simulink model "simulation_environment_thesis"  (R2024b)
settings: Solver=VariableStepAuto  StartTime=0.0  StopTime=3200  RelTol=1e-3
values from neighbouring files (symbols this model uses):
  A = [1, dt; 0, 1]    [estimate_and_control_fully_actuated_with_current.m]
  A1 = [1, 0, 0, 0, dt, 0 ... (first row only)    [estimate_and_control_fully_actuated_with_current.m]
  alpha = 10    [estimate_and_control_fully_actuated_with_current.m]
  alpha_xy_1 = 1    [estimate_and_control_fully_actuated_with_current.m]
  alpha_z_1 = 5    [estimate_and_control_fully_actuated_with_current.m]
  Az1 = [1, dt ... (first row only)    [estimate_and_control_fully_actuated_with_current.m]
  B = [dt; 0]    [estimate_and_control_fully_actuated_with_current.m]
  B1 = [dt, 0 ... (first row only)    [estimate_and_control_fully_actuated_with_current.m]
  Bz1 = [dt ... (first row only)    [estimate_and_control_fully_actuated_with_current.m]
  C1 = [1, 0, 0, 0, 0, 0 ... (first row only)    [estimate_and_control_fully_actuated_with_current.m]
  circumference_x = min_dist_from_ds*cos(theta)    [estimate_and_control_fully_actuated_with_current.m]
  circumference_y = min_dist_from_ds*sin(theta)    [estimate_and_control_fully_actuated_with_current.m]
  const_current = [-r; -s; 0; 0]    [estimate_and_control_fully_actuated_with_current.m]
  csi_psi_1 = 0.0003    [estimate_and_control_fully_actuated_with_current.m]
  csi_r_1 = 0.0003    [estimate_and_control_fully_actuated_with_current.m]
  csi_vcx_1 = 0.0003    [estimate_and_control_fully_actuated_with_current.m]
  csi_vcy_1 = 0.0003    [estimate_and_control_fully_actuated_with_current.m]
  csi_vcz_1 = 0.005    [estimate_and_control_fully_actuated_with_current.m]
  csi_x_1 = 0.003    [estimate_and_control_fully_actuated_with_current.m]
  csi_y_1 = 0.003    [estimate_and_control_fully_actuated_with_current.m]
  csi_z_1 = 0.005    [estimate_and_control_fully_actuated_with_current.m]
  Cz1 = [1, 0]    [estimate_and_control_fully_actuated_with_current.m]
  d = 1    [estimate_and_control_fully_actuated_with_current.m]
  delta_FOV = pi/7    (= 0.448798950513)    [estimate_and_control_fully_actuated_with_current.m]
  desired_speed = speed_0    [estimate_and_control_fully_actuated_with_current.m]
  dt = 1/Tsim    (= 0.005)    [estimate_and_control_fully_actuated_with_current.m]
  eta1 = deg2rad(0.5)    [estimate_and_control_fully_actuated_with_current.m]
  eta2 = deg2rad(0.5)    [estimate_and_control_fully_actuated_with_current.m]
  eta_psi_1 = 0.003    [estimate_and_control_fully_actuated_with_current.m]
  eta_x_1 = 0.03    [estimate_and_control_fully_actuated_with_current.m]
  eta_y_1 = 0.03    [estimate_and_control_fully_actuated_with_current.m]
  eta_z_1 = 0.005    [estimate_and_control_fully_actuated_with_current.m]
  G = dt*eye(2)    [estimate_and_control_fully_actuated_with_current.m]
  G1 = dt*eye(6)    [estimate_and_control_fully_actuated_with_current.m]
  Gz1 = dt*eye(2)    [estimate_and_control_fully_actuated_with_current.m]
  left_reference = L_x*[cos(NED_psi_ds); sin(NED_psi_ds); 0] + L_y_l*[-sin(NED_psi_ds); cos(NED_psi_ds); 0]    [estimate_and_control_fully_actuated_with_current.m]
  max_dist_from_ship = 60    [estimate_and_control_fully_actuated_with_current.m]
  min_dist_from_ds = 45    [estimate_and_control_fully_actuated_with_current.m]
  mu = -5    [estimate_and_control_fully_actuated_with_current.m]
  NED_psi_ds = -pi/2    (= -1.57079632679)    [estimate_and_control_fully_actuated_with_current.m]
  p_b1 = [-90; 90; 5]    [estimate_and_control_fully_actuated_with_current.m]
  position_t_0 = [0; 0; 0; 0]    [estimate_and_control_fully_actuated_with_current.m]
  psi_P = linspace(0,2*pi,360)'    [estimate_and_control_fully_actuated_with_current.m]
  Q = [csi1_kf^2, 0 ... (first row only)    [estimate_and_control_fully_actuated_with_current.m]
  Q1 = [csi_x_1_kf^2, 0, 0, 0, 0, 0 ... (first row only)    [estimate_and_control_fully_actuated_with_current.m]
  Qz1 = [csi_z_1_kf^2, 0 ... (first row only)    [estimate_and_control_fully_actuated_with_current.m]
  R = [eta1_kf^2, 0 ... (first row only)    [estimate_and_control_fully_actuated_with_current.m]
  R1 = [eta_x_1_kf^2, 0, 0 ... (first row only)    [estimate_and_control_fully_actuated_with_current.m]
  right_reference = L_x*[cos(NED_psi_ds); sin(NED_psi_ds); 0] + L_y_r*[sin(NED_psi_ds); -cos(NED_psi_ds); 0]    [estimate_and_control_fully_actuated_with_current.m]
  Rz1 = eta_z_1_kf^2    (= 1e-06)    [estimate_and_control_fully_actuated_with_current.m]
  speed_0 = [0.1, 0.1, 0, 0]'    [estimate_and_control_fully_actuated_with_current.m]
  tau_heave_sat = 30    [estimate_and_control_fully_actuated_with_current.m]
  tau_surge_sat = 10    [estimate_and_control_fully_actuated_with_current.m]
  tau_sway_sat = 30    [estimate_and_control_fully_actuated_with_current.m]
  tau_yaw_sat = 0.1    [estimate_and_control_fully_actuated_with_current.m]
  var_vm_x = 0.001    [estimate_and_control_fully_actuated_with_current.m]
  var_vm_y = 0.001    [estimate_and_control_fully_actuated_with_current.m]
  var_vm_z = 0.001    [estimate_and_control_fully_actuated_with_current.m]
undefined symbols (not defined in the file or in its folder): A2, alpha2, B2, csi1_2, csi2_2, csi3_2, csi4_2, csi5_2, csi6_2, csi7_2, csi8_2, eta1_2, eta2_2, eta3_2, eta4_2, eta5_2, eta6_2, G2, Q2, R2
libraries: simulink x60, simulink_extras x5
(part 3 of 16)

== system simulation_environment_thesis/toWorkspace blocks 24 ==
  state : Inport
  psi1 : Inport  Port=2
  body_speed_1 : Inport  Port=3
  psi2 : Inport  Port=4
  p1 : Inport  Port=5
  p2 : Inport  Port=6
  speed body2 : Inport  Port=7
  speed body3 : Inport  Port=8
  psi3 : Inport  Port=9
  p3 : Inport  Port=10
  speed_body4 : Inport  Port=11
  psi4 : Inport  Port=12
  p4 : Inport  Port=13
  Demux{,1..2,6} (4 alike) : Demux
  Demux{3..5,7} (4 alike) : Demux  Outputs=3
  Multiport Switch{,1..6} (7 alike) : MultiPortSwitch  Inputs=4  SaturateOnIntegerOverflow=off  InputSameDT=off
  To Workspace : ToWorkspace  VariableName=z  SampleTime=-1
  To Workspace1 : ToWorkspace  VariableName=w  SampleTime=-1
  To Workspace15 : ToWorkspace  VariableName=x  SampleTime=-1
  To Workspace16 : ToWorkspace  VariableName=y  SampleTime=-1
  To Workspace17 : ToWorkspace  VariableName=u  SampleTime=-1
  To Workspace18 : ToWorkspace  VariableName=v  SampleTime=-1
  To Workspace19 : ToWorkspace  VariableName=yaw  SampleTime=-1
  wires:
  Multiport Switch/1 -> To Workspace19/1
  Multiport Switch5/1 -> To Workspace16/1
  Multiport Switch2/1 -> To Workspace18/1
  Multiport Switch1/1 -> To Workspace17/1
  Multiport Switch6/1 -> To Workspace/1
  Multiport Switch3/1 -> To Workspace1/1
  psi1/1 -> Multiport Switch/2
  psi2/1 -> Multiport Switch/3
  psi3/1 -> Multiport Switch/4
  body_speed_1/1 -> Demux/1
  speed body2/1 -> Demux1/1
  speed body3/1 -> Demux2/1
  Demux/1 -> Multiport Switch1/2
  Demux1/1 -> Multiport Switch1/3
  Demux2/1 -> Multiport Switch1/4
  Demux/2 -> Multiport Switch2/2
  Demux1/2 -> Multiport Switch2/3
  Demux2/2 -> Multiport Switch2/4
  Demux/3 -> Multiport Switch3/2
  Demux1/3 -> Multiport Switch3/3
  Demux2/3 -> Multiport Switch3/4
  Demux3/1 -> Multiport Switch4/2
  Demux3/2 -> Multiport Switch5/2
  Demux4/2 -> Multiport Switch5/3
  p3/1 -> Demux5/1
  Demux5/2 -> Multiport Switch5/4
  Demux3/3 -> Multiport Switch6/2
  Demux5/1 -> Multiport Switch4/4
  Multiport Switch4/1 -> To Workspace15/1
  Demux4/3 -> Multiport Switch6/3
  state/1 -> Multiport Switch3/1, Multiport Switch2/1, Multiport Switch1/1, Multiport Switch/1, Multiport Switch6/1, Multiport Switch5/1, Multiport Switch4/1
  p1/1 -> Demux3/1
  p2/1 -> Demux4/1
  Demux4/1 -> Multiport Switch4/3
  Demux5/3 -> Multiport Switch6/4
  speed_body4/1 -> Demux6/1
  psi4/1 -> Multiport Switch/5
  p4/1 -> Demux7/1
  Demux6/1 -> Multiport Switch1/5
  Demux6/2 -> Multiport Switch2/5
  Demux6/3 -> Multiport Switch3/5
  Demux7/1 -> Multiport Switch4/5
  Demux7/2 -> Multiport Switch5/5
  Demux7/3 -> Multiport Switch6/5

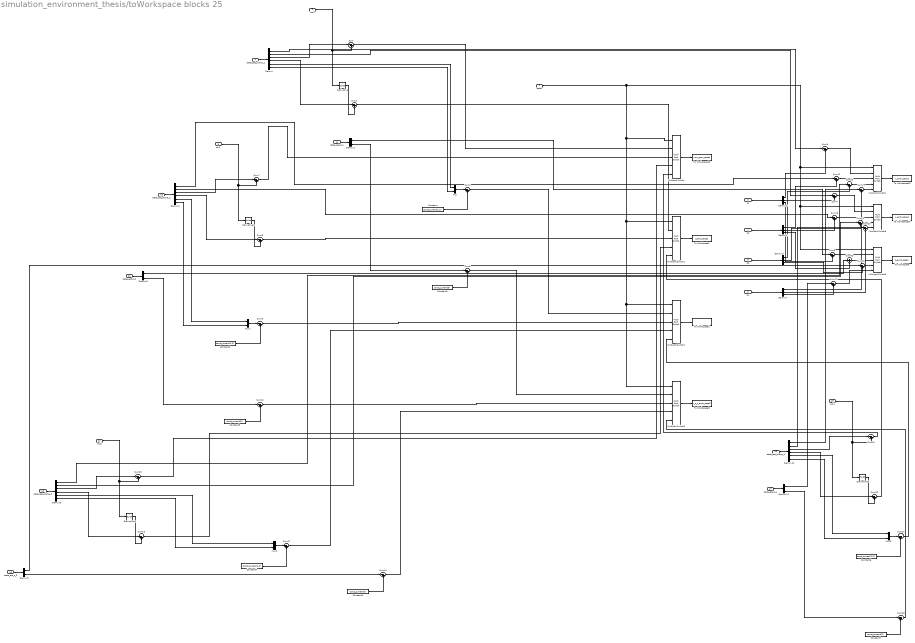
== system simulation_environment_thesis/toWorkspace blocks 25 ==
  state : Inport
  psi1 : Inport  Port=2
  p1 : Inport  Port=3
  p2 : Inport  Port=4
  p3 : Inport  Port=5
  p4 : Inport  Port=6
  state_est_plane_1 : Inport  Port=7
  state_est_z_1 : Inport  Port=8
  psi2 : Inport  Port=9
  state_est_plane_2 : Inport  Port=10
  state_est_z_2 : Inport  Port=11
  psi3 : Inport  Port=12
  state_est_plane_3 : Inport  Port=13
  state_est_z_3 : Inport  Port=14
  psi4 : Inport  Port=15
  state_est_plane_4 : Inport  Port=16
  state_est_z_4 : Inport  Port=17
  Constant{,2,4,6} (4 alike) : Constant  Value=const_current(1:2)
  Constant{1,3,5,7} (4 alike) : Constant  Value=const_current(3)
  Demux{,2,8,10} (4 alike) : Demux  Outputs=6
  Demux{1,6,9,11} (4 alike) : Demux  Outputs=2
  Demux{3..5,7} (4 alike) : Demux  Outputs=3
  Derivative{,1..3} (4 alike) : Derivative
  Multiport Switch{,1..6} (7 alike) : MultiPortSwitch  Inputs=4  SaturateOnIntegerOverflow=off  InputSameDT=off
  Mux{,1..3} (4 alike) : Mux  Inputs=2
  Sum{,1..27} (28 alike) : Sum  Inputs=|-+
  To Workspace : ToWorkspace  VariableName=z_error_case1  SampleTime=-1
  To Workspace1 : ToWorkspace  VariableName=r_error_case1  SampleTime=-1
  To Workspace15 : ToWorkspace  VariableName=x_error_case1  SampleTime=-1
  To Workspace16 : ToWorkspace  VariableName=y_error_case1  SampleTime=-1
  To Workspace19 : ToWorkspace  VariableName=yaw_error_case1  SampleTime=-1
  To Workspace2 : ToWorkspace  VariableName=v_c_x_y_error_case1  SampleTime=-1
  To Workspace3 : ToWorkspace  VariableName=v_c_z_error_case1  SampleTime=-1
  wires:
  Multiport Switch/1 -> To Workspace19/1
  Multiport Switch5/1 -> To Workspace16/1
  Multiport Switch6/1 -> To Workspace/1
  Demux3/1 -> Sum3/2
  p3/1 -> Demux5/1
  Multiport Switch4/1 -> To Workspace15/1
  p1/1 -> Demux3/1
  p2/1 -> Demux4/1
  p4/1 -> Demux7/1
  state_est_plane_1/1 -> Demux/1
  Demux/3 -> Sum/1
  psi1/1 -> Derivative/1, Sum/2
  Sum/1 -> Multiport Switch/2
  Multiport Switch1/1 -> To Workspace1/1
  Derivative/1 -> Sum1/2
  Demux/4 -> Sum1/1
  Sum1/1 -> Multiport Switch1/2
  Sum2/1 -> Multiport Switch2/2
  Multiport Switch2/1 -> To Workspace2/1
  Mux/1 -> Sum2/1
  Demux/5 -> Mux/1
  Demux/6 -> Mux/2
  Constant/1 -> Sum2/2
  Demux/1 -> Sum3/1
  Sum3/1 -> Multiport Switch4/2
  Demux3/2 -> Sum4/2
  Demux/2 -> Sum4/1
  Sum4/1 -> Multiport Switch5/2
  Demux3/3 -> Sum5/2
  state_est_z_1/1 -> Demux1/1
  Demux1/1 -> Sum5/1
  Sum5/1 -> Multiport Switch6/2
  Multiport Switch3/1 -> To Workspace3/1
  Sum6/1 -> Multiport Switch3/2
  state/1 -> Multiport Switch1/1, Multiport Switch3/1, Multiport Switch2/1, Multiport Switch/1, Multiport Switch4/1, Multiport Switch6/1, Multiport Switch5/1
  Constant1/1 -> Sum6/2
  Demux1/2 -> Sum6/1
  Derivative1/1 -> Sum8/2
  Demux6/2 -> Sum10/1
  Demux2/5 -> Mux1/1
  Demux2/4 -> Sum8/1
  Constant3/1 -> Sum10/2
  Demux2/1 -> Sum11/1
  state_est_z_2/1 -> Demux6/1
  Sum7/1 -> Multiport Switch/3
  Demux2/3 -> Sum7/1
  Demux6/1 -> Sum13/1
  Demux2/6 -> Mux1/2
  psi2/1 -> Derivative1/1, Sum7/2
  Constant2/1 -> Sum9/2
  Sum9/1 -> Multiport Switch2/3
  Sum10/1 -> Multiport Switch3/3
  Sum8/1 -> Multiport Switch1/3
  Mux1/1 -> Sum9/1
  state_est_plane_2/1 -> Demux2/1
  Demux2/2 -> Sum12/1
  Sum11/1 -> Multiport Switch4/3
  Demux4/1 -> Sum11/2
  Sum12/1 -> Multiport Switch5/3
  Demux4/2 -> Sum12/2
  Demux4/3 -> Sum13/2
  Sum13/1 -> Multiport Switch6/3
  Constant5/1 -> Sum14/2
  Demux8/4 -> Sum16/1
  Demux9/2 -> Sum14/1
  Demux8/5 -> Mux2/1
  Derivative2/1 -> Sum16/2
  state_est_plane_3/1 -> Demux8/1
  Mux2/1 -> Sum17/1
  Demux8/6 -> Mux2/2
  Constant4/1 -> Sum17/2
  psi3/1 -> Derivative2/1, Sum15/2
  state_est_z_3/1 -> Demux9/1
  Demux8/3 -> Sum15/1
  Demux10/3 -> Sum19/1
  state_est_z_4/1 -> Demux11/1
  psi4/1 -> Derivative3/1, Sum19/2
  Demux10/6 -> Mux3/2
  state_est_plane_4/1 -> Demux10/1
  Constant6/1 -> Sum21/2
  Mux3/1 -> Sum21/1
  Derivative3/1 -> Sum20/2
  Demux10/4 -> Sum20/1
  Demux11/2 -> Sum18/1
  Demux10/5 -> Mux3/1
  Constant7/1 -> Sum18/2
  Sum15/1 -> Multiport Switch/4
  Sum16/1 -> Multiport Switch1/4
  Sum17/1 -> Multiport Switch2/4
  Sum14/1 -> Multiport Switch3/4
  Sum19/1 -> Multiport Switch/5
  Sum20/1 -> Multiport Switch1/5
  Sum21/1 -> Multiport Switch2/5
  Sum18/1 -> Multiport Switch3/5
  Sum22/1 -> Multiport Switch4/4
  Demux5/1 -> Sum22/2
  Demux8/1 -> Sum22/1
  Sum23/1 -> Multiport Switch4/5
  Demux7/1 -> Sum23/2
  Demux10/1 -> Sum23/1
  Sum24/1 -> Multiport Switch5/4
  Demux5/2 -> Sum24/2
  Demux8/2 -> Sum24/1
  Sum25/1 -> Multiport Switch5/5
  Demux7/2 -> Sum25/2
  Demux10/2 -> Sum25/1
  Sum26/1 -> Multiport Switch6/4
  Demux5/3 -> Sum26/2
  Demux9/1 -> Sum26/1
  Sum27/1 -> Multiport Switch6/5
  Demux7/3 -> Sum27/2
  Demux11/1 -> Sum27/1

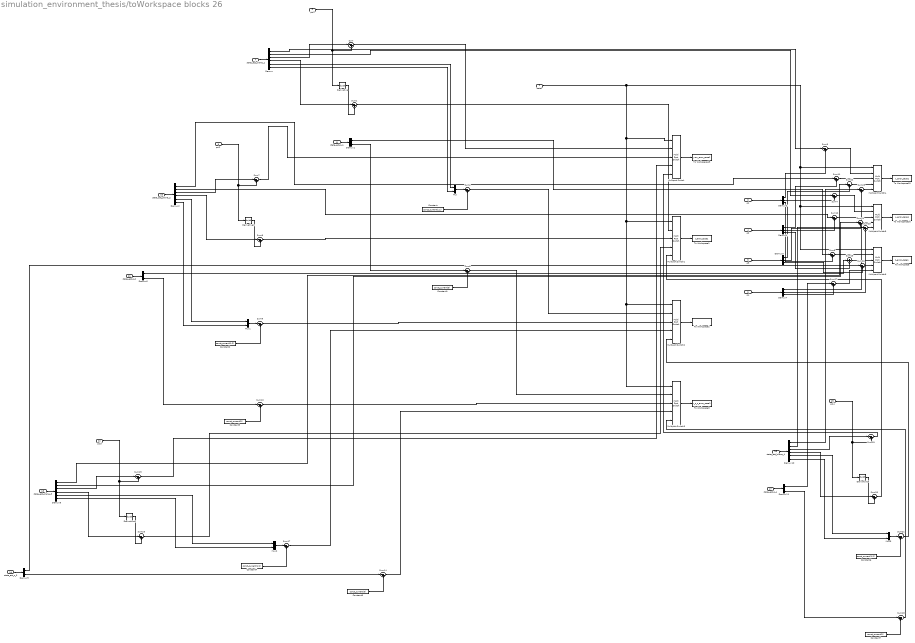
== system simulation_environment_thesis/toWorkspace blocks 26 ==
  state : Inport
  psi1 : Inport  Port=2
  p1 : Inport  Port=3
  p2 : Inport  Port=4
  p3 : Inport  Port=5
  p4 : Inport  Port=6
  state_est_plane_1 : Inport  Port=7
  state_est_z_1 : Inport  Port=8
  psi2 : Inport  Port=9
  state_est_plane_2 : Inport  Port=10
  state_est_z_2 : Inport  Port=11
  psi3 : Inport  Port=12
  state_est_plane_3 : Inport  Port=13
  state_est_z_3 : Inport  Port=14
  psi4 : Inport  Port=15
  state_est_plane_4 : Inport  Port=16
  state_est_z_4 : Inport  Port=17
  Constant{,2,4,6} (4 alike) : Constant  Value=const_current(1:2)
  Constant{1,3,5,7} (4 alike) : Constant  Value=const_current(3)
  Demux{,2,8,10} (4 alike) : Demux  Outputs=6
  Demux{1,6,9,11} (4 alike) : Demux  Outputs=2
  Demux{3..5,7} (4 alike) : Demux  Outputs=3
  Derivative{,1..3} (4 alike) : Derivative
  Multiport Switch{,1..6} (7 alike) : MultiPortSwitch  Inputs=4  SaturateOnIntegerOverflow=off  InputSameDT=off
  Mux{,1..3} (4 alike) : Mux  Inputs=2
  Sum{,1..27} (28 alike) : Sum  Inputs=|-+
  To Workspace : ToWorkspace  VariableName=z_error_case1  SampleTime=-1
  To Workspace1 : ToWorkspace  VariableName=r_error_case1  SampleTime=-1
  To Workspace15 : ToWorkspace  VariableName=x_error_case1  SampleTime=-1
  To Workspace16 : ToWorkspace  VariableName=y_error_case1  SampleTime=-1
  To Workspace19 : ToWorkspace  VariableName=yaw_error_case1  SampleTime=-1
  To Workspace2 : ToWorkspace  VariableName=v_c_x_y_error_case1  SampleTime=-1
  To Workspace3 : ToWorkspace  VariableName=v_c_z_error_case1  SampleTime=-1
  wires:
  Multiport Switch/1 -> To Workspace19/1
  Multiport Switch5/1 -> To Workspace16/1
  Multiport Switch6/1 -> To Workspace/1
  Demux3/1 -> Sum3/2
  p3/1 -> Demux5/1
  Multiport Switch4/1 -> To Workspace15/1
  p1/1 -> Demux3/1
  p2/1 -> Demux4/1
  p4/1 -> Demux7/1
  state_est_plane_1/1 -> Demux/1
  Demux/3 -> Sum/1
  psi1/1 -> Derivative/1, Sum/2
  Sum/1 -> Multiport Switch/2
  Multiport Switch1/1 -> To Workspace1/1
  Derivative/1 -> Sum1/2
  Demux/4 -> Sum1/1
  Sum1/1 -> Multiport Switch1/2
  Sum2/1 -> Multiport Switch2/2
  Multiport Switch2/1 -> To Workspace2/1
  Mux/1 -> Sum2/1
  Demux/5 -> Mux/1
  Demux/6 -> Mux/2
  Constant/1 -> Sum2/2
  Demux/1 -> Sum3/1
  Sum3/1 -> Multiport Switch4/2
  Demux3/2 -> Sum4/2
  Demux/2 -> Sum4/1
  Sum4/1 -> Multiport Switch5/2
  Demux3/3 -> Sum5/2
  state_est_z_1/1 -> Demux1/1
  Demux1/1 -> Sum5/1
  Sum5/1 -> Multiport Switch6/2
  Multiport Switch3/1 -> To Workspace3/1
  Sum6/1 -> Multiport Switch3/2
  state/1 -> Multiport Switch1/1, Multiport Switch3/1, Multiport Switch2/1, Multiport Switch/1, Multiport Switch4/1, Multiport Switch6/1, Multiport Switch5/1
  Constant1/1 -> Sum6/2
  Demux1/2 -> Sum6/1
  Derivative1/1 -> Sum8/2
  Demux6/2 -> Sum10/1
  Demux2/5 -> Mux1/1
  Demux2/4 -> Sum8/1
  Constant3/1 -> Sum10/2
  Demux2/1 -> Sum11/1
  state_est_z_2/1 -> Demux6/1
  Sum7/1 -> Multiport Switch/3
  Demux2/3 -> Sum7/1
  Demux6/1 -> Sum13/1
  Demux2/6 -> Mux1/2
  psi2/1 -> Derivative1/1, Sum7/2
  Constant2/1 -> Sum9/2
  Sum9/1 -> Multiport Switch2/3
  Sum10/1 -> Multiport Switch3/3
  Sum8/1 -> Multiport Switch1/3
  Mux1/1 -> Sum9/1
  state_est_plane_2/1 -> Demux2/1
  Demux2/2 -> Sum12/1
  Sum11/1 -> Multiport Switch4/3
  Demux4/1 -> Sum11/2
  Sum12/1 -> Multiport Switch5/3
  Demux4/2 -> Sum12/2
  Demux4/3 -> Sum13/2
  Sum13/1 -> Multiport Switch6/3
  Constant5/1 -> Sum14/2
  Demux8/4 -> Sum16/1
  Demux9/2 -> Sum14/1
  Demux8/5 -> Mux2/1
  Derivative2/1 -> Sum16/2
  state_est_plane_3/1 -> Demux8/1
  Mux2/1 -> Sum17/1
  Demux8/6 -> Mux2/2
  Constant4/1 -> Sum17/2
  psi3/1 -> Derivative2/1, Sum15/2
  state_est_z_3/1 -> Demux9/1
  Demux8/3 -> Sum15/1
  Demux10/3 -> Sum19/1
  state_est_z_4/1 -> Demux11/1
  psi4/1 -> Derivative3/1, Sum19/2
  Demux10/6 -> Mux3/2
  state_est_plane_4/1 -> Demux10/1
  Constant6/1 -> Sum21/2
  Mux3/1 -> Sum21/1
  Derivative3/1 -> Sum20/2
  Demux10/4 -> Sum20/1
  Demux11/2 -> Sum18/1
  Demux10/5 -> Mux3/1
  Constant7/1 -> Sum18/2
  Sum15/1 -> Multiport Switch/4
  Sum16/1 -> Multiport Switch1/4
  Sum17/1 -> Multiport Switch2/4
  Sum14/1 -> Multiport Switch3/4
  Sum19/1 -> Multiport Switch/5
  Sum20/1 -> Multiport Switch1/5
  Sum21/1 -> Multiport Switch2/5
  Sum18/1 -> Multiport Switch3/5
  Sum22/1 -> Multiport Switch4/4
  Demux5/1 -> Sum22/2
  Demux8/1 -> Sum22/1
  Sum23/1 -> Multiport Switch4/5
  Demux7/1 -> Sum23/2
  Demux10/1 -> Sum23/1
  Sum24/1 -> Multiport Switch5/4
  Demux5/2 -> Sum24/2
  Demux8/2 -> Sum24/1
  Sum25/1 -> Multiport Switch5/5
  Demux7/2 -> Sum25/2
  Demux10/2 -> Sum25/1
  Sum26/1 -> Multiport Switch6/4
  Demux5/3 -> Sum26/2
  Demux9/1 -> Sum26/1
  Sum27/1 -> Multiport Switch6/5
  Demux7/3 -> Sum27/2
  Demux11/1 -> Sum27/1

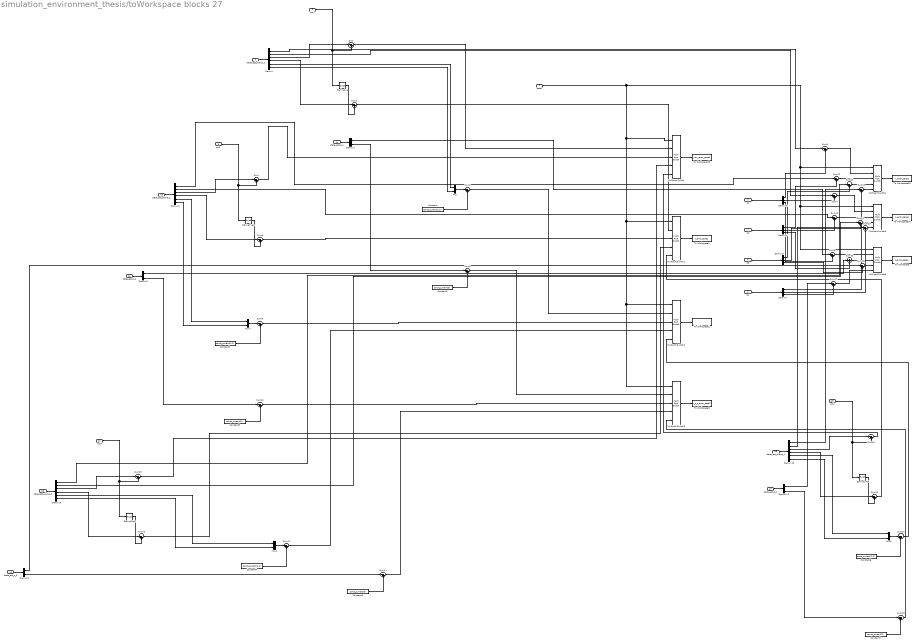
== system simulation_environment_thesis/toWorkspace blocks 27 ==
  state : Inport
  psi1 : Inport  Port=2
  p1 : Inport  Port=3
  p2 : Inport  Port=4
  p3 : Inport  Port=5
  p4 : Inport  Port=6
  state_est_plane_1 : Inport  Port=7
  state_est_z_1 : Inport  Port=8
  psi2 : Inport  Port=9
  state_est_plane_2 : Inport  Port=10
  state_est_z_2 : Inport  Port=11
  psi3 : Inport  Port=12
  state_est_plane_3 : Inport  Port=13
  state_est_z_3 : Inport  Port=14
  psi4 : Inport  Port=15
  state_est_plane_4 : Inport  Port=16
  state_est_z_4 : Inport  Port=17
  Constant{,2,4,6} (4 alike) : Constant  Value=const_current(1:2)
  Constant{1,3,5,7} (4 alike) : Constant  Value=const_current(3)
  Demux{,2,8,10} (4 alike) : Demux  Outputs=6
  Demux{1,6,9,11} (4 alike) : Demux  Outputs=2
  Demux{3..5,7} (4 alike) : Demux  Outputs=3
  Derivative{,1..3} (4 alike) : Derivative
  Multiport Switch{,1..6} (7 alike) : MultiPortSwitch  Inputs=4  SaturateOnIntegerOverflow=off  InputSameDT=off
  Mux{,1..3} (4 alike) : Mux  Inputs=2
  Sum{,1..27} (28 alike) : Sum  Inputs=|-+
  To Workspace : ToWorkspace  VariableName=z_error_case1  SampleTime=-1
  To Workspace1 : ToWorkspace  VariableName=r_error_case1  SampleTime=-1
  To Workspace15 : ToWorkspace  VariableName=x_error_case1  SampleTime=-1
  To Workspace16 : ToWorkspace  VariableName=y_error_case1  SampleTime=-1
  To Workspace19 : ToWorkspace  VariableName=yaw_error_case1  SampleTime=-1
  To Workspace2 : ToWorkspace  VariableName=v_c_x_y_error_case1  SampleTime=-1
  To Workspace3 : ToWorkspace  VariableName=v_c_z_error_case1  SampleTime=-1
  wires:
  Multiport Switch/1 -> To Workspace19/1
  Multiport Switch5/1 -> To Workspace16/1
  Multiport Switch6/1 -> To Workspace/1
  Demux3/1 -> Sum3/2
  p3/1 -> Demux5/1
  Multiport Switch4/1 -> To Workspace15/1
  p1/1 -> Demux3/1
  p2/1 -> Demux4/1
  p4/1 -> Demux7/1
  state_est_plane_1/1 -> Demux/1
  Demux/3 -> Sum/1
  psi1/1 -> Derivative/1, Sum/2
  Sum/1 -> Multiport Switch/2
  Multiport Switch1/1 -> To Workspace1/1
  Derivative/1 -> Sum1/2
  Demux/4 -> Sum1/1
  Sum1/1 -> Multiport Switch1/2
  Sum2/1 -> Multiport Switch2/2
  Multiport Switch2/1 -> To Workspace2/1
  Mux/1 -> Sum2/1
  Demux/5 -> Mux/1
  Demux/6 -> Mux/2
  Constant/1 -> Sum2/2
  Demux/1 -> Sum3/1
  Sum3/1 -> Multiport Switch4/2
  Demux3/2 -> Sum4/2
  Demux/2 -> Sum4/1
  Sum4/1 -> Multiport Switch5/2
  Demux3/3 -> Sum5/2
  state_est_z_1/1 -> Demux1/1
  Demux1/1 -> Sum5/1
  Sum5/1 -> Multiport Switch6/2
  Multiport Switch3/1 -> To Workspace3/1
  Sum6/1 -> Multiport Switch3/2
  state/1 -> Multiport Switch1/1, Multiport Switch3/1, Multiport Switch2/1, Multiport Switch/1, Multiport Switch4/1, Multiport Switch6/1, Multiport Switch5/1
  Constant1/1 -> Sum6/2
  Demux1/2 -> Sum6/1
  Derivative1/1 -> Sum8/2
  Demux6/2 -> Sum10/1
  Demux2/5 -> Mux1/1
  Demux2/4 -> Sum8/1
  Constant3/1 -> Sum10/2
  Demux2/1 -> Sum11/1
  state_est_z_2/1 -> Demux6/1
  Sum7/1 -> Multiport Switch/3
  Demux2/3 -> Sum7/1
  Demux6/1 -> Sum13/1
  Demux2/6 -> Mux1/2
  psi2/1 -> Derivative1/1, Sum7/2
  Constant2/1 -> Sum9/2
  Sum9/1 -> Multiport Switch2/3
  Sum10/1 -> Multiport Switch3/3
  Sum8/1 -> Multiport Switch1/3
  Mux1/1 -> Sum9/1
  state_est_plane_2/1 -> Demux2/1
  Demux2/2 -> Sum12/1
  Sum11/1 -> Multiport Switch4/3
  Demux4/1 -> Sum11/2
  Sum12/1 -> Multiport Switch5/3
  Demux4/2 -> Sum12/2
  Demux4/3 -> Sum13/2
  Sum13/1 -> Multiport Switch6/3
  Constant5/1 -> Sum14/2
  Demux8/4 -> Sum16/1
  Demux9/2 -> Sum14/1
  Demux8/5 -> Mux2/1
  Derivative2/1 -> Sum16/2
  state_est_plane_3/1 -> Demux8/1
  Mux2/1 -> Sum17/1
  Demux8/6 -> Mux2/2
  Constant4/1 -> Sum17/2
  psi3/1 -> Derivative2/1, Sum15/2
  state_est_z_3/1 -> Demux9/1
  Demux8/3 -> Sum15/1
  Demux10/3 -> Sum19/1
  state_est_z_4/1 -> Demux11/1
  psi4/1 -> Derivative3/1, Sum19/2
  Demux10/6 -> Mux3/2
  state_est_plane_4/1 -> Demux10/1
  Constant6/1 -> Sum21/2
  Mux3/1 -> Sum21/1
  Derivative3/1 -> Sum20/2
  Demux10/4 -> Sum20/1
  Demux11/2 -> Sum18/1
  Demux10/5 -> Mux3/1
  Constant7/1 -> Sum18/2
  Sum15/1 -> Multiport Switch/4
  Sum16/1 -> Multiport Switch1/4
  Sum17/1 -> Multiport Switch2/4
  Sum14/1 -> Multiport Switch3/4
  Sum19/1 -> Multiport Switch/5
  Sum20/1 -> Multiport Switch1/5
  Sum21/1 -> Multiport Switch2/5
  Sum18/1 -> Multiport Switch3/5
  Sum22/1 -> Multiport Switch4/4
  Demux5/1 -> Sum22/2
  Demux8/1 -> Sum22/1
  Sum23/1 -> Multiport Switch4/5
  Demux7/1 -> Sum23/2
  Demux10/1 -> Sum23/1
  Sum24/1 -> Multiport Switch5/4
  Demux5/2 -> Sum24/2
  Demux8/2 -> Sum24/1
  Sum25/1 -> Multiport Switch5/5
  Demux7/2 -> Sum25/2
  Demux10/2 -> Sum25/1
  Sum26/1 -> Multiport Switch6/4
  Demux5/3 -> Sum26/2
  Demux9/1 -> Sum26/1
  Sum27/1 -> Multiport Switch6/5
  Demux7/3 -> Sum27/2
  Demux11/1 -> Sum27/1

== system simulation_environment_thesis/toWorkspace blocks 29 ==
  state : Inport
  psi1 : Inport  Port=2
  body_speed_1 : Inport  Port=3
  psi2 : Inport  Port=4
  p1 : Inport  Port=5
  p2 : Inport  Port=6
  speed body2 : Inport  Port=7
  speed body3 : Inport  Port=8
  psi3 : Inport  Port=9
  p3 : Inport  Port=10
  speed_body4 : Inport  Port=11
  psi4 : Inport  Port=12
  p4 : Inport  Port=13
  Demux{,1..2,6} (4 alike) : Demux
  Multiport Switch{,1..4,6} (6 alike) : MultiPortSwitch  Inputs=4  SaturateOnIntegerOverflow=off  InputSameDT=off
  To Workspace : ToWorkspace  VariableName=z_error  SampleTime=-1
  To Workspace1 : ToWorkspace  VariableName=w_error  SampleTime=-1
  To Workspace17 : ToWorkspace  VariableName=u_error  SampleTime=-1
  To Workspace18 : ToWorkspace  VariableName=v_error  SampleTime=-1
  To Workspace19 : ToWorkspace  VariableName=yaw_error  SampleTime=-1
  To Workspace2 : ToWorkspace  VariableName=r_error  SampleTime=-1
  wires:
  Multiport Switch/1 -> To Workspace19/1
  Multiport Switch2/1 -> To Workspace18/1
  Multiport Switch1/1 -> To Workspace17/1
  Multiport Switch6/1 -> To Workspace/1
  Multiport Switch3/1 -> To Workspace1/1
  psi1/1 -> Multiport Switch/2
  psi2/1 -> Multiport Switch/3
  psi3/1 -> Multiport Switch/4
  body_speed_1/1 -> Demux/1
  speed body2/1 -> Demux1/1
  speed body3/1 -> Demux2/1
  Demux/1 -> Multiport Switch1/2
  Demux1/1 -> Multiport Switch1/3
  Demux2/1 -> Multiport Switch1/4
  Demux/2 -> Multiport Switch2/2
  Demux1/2 -> Multiport Switch2/3
  Demux2/2 -> Multiport Switch2/4
  Demux/3 -> Multiport Switch3/2
  Demux1/3 -> Multiport Switch3/3
  Demux2/3 -> Multiport Switch3/4
  p1/1 -> Multiport Switch6/2
  p2/1 -> Multiport Switch6/3
  state/1 -> Multiport Switch4/1, Multiport Switch3/1, Multiport Switch2/1, Multiport Switch1/1, Multiport Switch/1, Multiport Switch6/1
  p3/1 -> Multiport Switch6/4
  speed_body4/1 -> Demux6/1
  psi4/1 -> Multiport Switch/5
  Demux6/1 -> Multiport Switch1/5
  Demux6/2 -> Multiport Switch2/5
  Demux6/3 -> Multiport Switch3/5
  p4/1 -> Multiport Switch6/5
  Multiport Switch4/1 -> To Workspace2/1
  Demux/4 -> Multiport Switch4/2
  Demux1/4 -> Multiport Switch4/3
  Demux2/4 -> Multiport Switch4/4
  Demux6/4 -> Multiport Switch4/5

== system simulation_environment_thesis/toWorkspace blocks 30 ==
  state : Inport
  psi1 : Inport  Port=2
  body_speed_1 : Inport  Port=3
  psi2 : Inport  Port=4
  p1 : Inport  Port=5
  p2 : Inport  Port=6
  speed body2 : Inport  Port=7
  speed body3 : Inport  Port=8
  psi3 : Inport  Port=9
  p3 : Inport  Port=10
  speed_body4 : Inport  Port=11
  psi4 : Inport  Port=12
  p4 : Inport  Port=13
  Demux{,1..2,6} (4 alike) : Demux
  Multiport Switch{,1..4,6} (6 alike) : MultiPortSwitch  Inputs=4  SaturateOnIntegerOverflow=off  InputSameDT=off
  To Workspace : ToWorkspace  VariableName=z_error  SampleTime=-1
  To Workspace1 : ToWorkspace  VariableName=w_error  SampleTime=-1
  To Workspace17 : ToWorkspace  VariableName=u_error  SampleTime=-1
  To Workspace18 : ToWorkspace  VariableName=v_error  SampleTime=-1
  To Workspace19 : ToWorkspace  VariableName=yaw_error  SampleTime=-1
  To Workspace2 : ToWorkspace  VariableName=r_error  SampleTime=-1
  wires:
  Multiport Switch/1 -> To Workspace19/1
  Multiport Switch2/1 -> To Workspace18/1
  Multiport Switch1/1 -> To Workspace17/1
  Multiport Switch6/1 -> To Workspace/1
  Multiport Switch3/1 -> To Workspace1/1
  psi1/1 -> Multiport Switch/2
  psi2/1 -> Multiport Switch/3
  psi3/1 -> Multiport Switch/4
  body_speed_1/1 -> Demux/1
  speed body2/1 -> Demux1/1
  speed body3/1 -> Demux2/1
  Demux/1 -> Multiport Switch1/2
  Demux1/1 -> Multiport Switch1/3
  Demux2/1 -> Multiport Switch1/4
  Demux/2 -> Multiport Switch2/2
  Demux1/2 -> Multiport Switch2/3
  Demux2/2 -> Multiport Switch2/4
  Demux/3 -> Multiport Switch3/2
  Demux1/3 -> Multiport Switch3/3
  Demux2/3 -> Multiport Switch3/4
  p1/1 -> Multiport Switch6/2
  p2/1 -> Multiport Switch6/3
  state/1 -> Multiport Switch4/1, Multiport Switch3/1, Multiport Switch2/1, Multiport Switch1/1, Multiport Switch/1, Multiport Switch6/1
  p3/1 -> Multiport Switch6/4
  speed_body4/1 -> Demux6/1
  psi4/1 -> Multiport Switch/5
  Demux6/1 -> Multiport Switch1/5
  Demux6/2 -> Multiport Switch2/5
  Demux6/3 -> Multiport Switch3/5
  p4/1 -> Multiport Switch6/5
  Multiport Switch4/1 -> To Workspace2/1
  Demux/4 -> Multiport Switch4/2
  Demux1/4 -> Multiport Switch4/3
  Demux2/4 -> Multiport Switch4/4
  Demux6/4 -> Multiport Switch4/5

== system simulation_environment_thesis/toWorkspace blocks 31 ==
  state : Inport
  psi1 : Inport  Port=2
  body_speed_1 : Inport  Port=3
  psi2 : Inport  Port=4
  p1 : Inport  Port=5
  p2 : Inport  Port=6
  speed body2 : Inport  Port=7
  speed body3 : Inport  Port=8
  psi3 : Inport  Port=9
  p3 : Inport  Port=10
  speed_body4 : Inport  Port=11
  psi4 : Inport  Port=12
  p4 : Inport  Port=13
  Demux{,1..2,6} (4 alike) : Demux
  Multiport Switch{,1..4,6} (6 alike) : MultiPortSwitch  Inputs=4  SaturateOnIntegerOverflow=off  InputSameDT=off
  To Workspace : ToWorkspace  VariableName=z_error  SampleTime=-1
  To Workspace1 : ToWorkspace  VariableName=w_error  SampleTime=-1
  To Workspace17 : ToWorkspace  VariableName=u_error  SampleTime=-1
  To Workspace18 : ToWorkspace  VariableName=v_error  SampleTime=-1
  To Workspace19 : ToWorkspace  VariableName=yaw_error  SampleTime=-1
  To Workspace2 : ToWorkspace  VariableName=r_error  SampleTime=-1
  wires:
  Multiport Switch/1 -> To Workspace19/1
  Multiport Switch2/1 -> To Workspace18/1
  Multiport Switch1/1 -> To Workspace17/1
  Multiport Switch6/1 -> To Workspace/1
  Multiport Switch3/1 -> To Workspace1/1
  psi1/1 -> Multiport Switch/2
  psi2/1 -> Multiport Switch/3
  psi3/1 -> Multiport Switch/4
  body_speed_1/1 -> Demux/1
  speed body2/1 -> Demux1/1
  speed body3/1 -> Demux2/1
  Demux/1 -> Multiport Switch1/2
  Demux1/1 -> Multiport Switch1/3
  Demux2/1 -> Multiport Switch1/4
  Demux/2 -> Multiport Switch2/2
  Demux1/2 -> Multiport Switch2/3
  Demux2/2 -> Multiport Switch2/4
  Demux/3 -> Multiport Switch3/2
  Demux1/3 -> Multiport Switch3/3
  Demux2/3 -> Multiport Switch3/4
  p1/1 -> Multiport Switch6/2
  p2/1 -> Multiport Switch6/3
  state/1 -> Multiport Switch4/1, Multiport Switch3/1, Multiport Switch2/1, Multiport Switch1/1, Multiport Switch/1, Multiport Switch6/1
  p3/1 -> Multiport Switch6/4
  speed_body4/1 -> Demux6/1
  psi4/1 -> Multiport Switch/5
  Demux6/1 -> Multiport Switch1/5
  Demux6/2 -> Multiport Switch2/5
  Demux6/3 -> Multiport Switch3/5
  p4/1 -> Multiport Switch6/5
  Multiport Switch4/1 -> To Workspace2/1
  Demux/4 -> Multiport Switch4/2
  Demux1/4 -> Multiport Switch4/3
  Demux2/4 -> Multiport Switch4/4
  Demux6/4 -> Multiport Switch4/5

== system simulation_environment_thesis/PHASE 1  /CONTROLLER1 ==
  v_err : Inport
  speed_0 : Inport  Port=2  PortDimensions=[4,1]
  yaw_err : Inport  Port=3
  z_err : Inport  Port=4
  ANTIWINDUP SURGE : Subsystem
  ANTIWINDUP SWAY : Subsystem
  ANTIWINDUP_HEAVE : Subsystem
  ANTIWINDUP_YAW : Subsystem
  Demux : Demux
  MATLAB Function3 : MATLAB Function  TreatAsAtomicUnit=on
  Mux : Mux
  tau : Outport
  wires:
  ANTIWINDUP SURGE/1(tau_u) -> Mux/1
  ANTIWINDUP_HEAVE/1(tau_w) -> Mux/3
  MATLAB Function3/1(K_i_u) -> ANTIWINDUP SURGE/2(K_int)
  MATLAB Function3/3(K_t_u) -> ANTIWINDUP SURGE/4(K_t_u)
  MATLAB Function3/2(K_p_u) -> ANTIWINDUP SURGE/3(K_prop)
  speed_0/1 -> MATLAB Function3/1(speed_0)
  Demux/1 -> ANTIWINDUP SURGE/1(in)
  ANTIWINDUP SWAY/1(tau_v) -> Mux/2
  v_err/1 -> Demux/1
  Mux/1 -> tau/1
  Demux/2 -> ANTIWINDUP SWAY/1(in)
  MATLAB Function3/4(K_i_v) -> ANTIWINDUP SWAY/2(K_int)
  MATLAB Function3/5(K_p_v) -> ANTIWINDUP SWAY/3(K_prop)
  MATLAB Function3/6(K_t_v) -> ANTIWINDUP SWAY/4(K_t_v)
  ANTIWINDUP_YAW/1(tau_psi) -> Mux/4
  MATLAB Function3/14(K_t_psi) -> ANTIWINDUP_YAW/3(K_t_psi)
  MATLAB Function3/13(K_d_psi) -> ANTIWINDUP_YAW/6(K_der)
  Demux/4 -> ANTIWINDUP_YAW/5(in)
  MATLAB Function3/12(K_p_psi) -> ANTIWINDUP_YAW/2(K_prop)
  MATLAB Function3/11(K_i_psi) -> ANTIWINDUP_YAW/1(K_int)
  yaw_err/1 -> ANTIWINDUP_YAW/4(in1)
  MATLAB Function3/7(K_i_w) -> ANTIWINDUP_HEAVE/1(K_int)
  MATLAB Function3/8(K_p_w) -> ANTIWINDUP_HEAVE/2(K_prop)
  MATLAB Function3/10(K_t_w) -> ANTIWINDUP_HEAVE/3(K_t_w)
  MATLAB Function3/9(K_d_w) -> ANTIWINDUP_HEAVE/6(K_der)
  z_err/1 -> ANTIWINDUP_HEAVE/4(z_err)
  Demux/3 -> ANTIWINDUP_HEAVE/5(w_err)

== system simulation_environment_thesis/PHASE 1  /REFERENCE GENERATOR ==
  p : Inport
  v_c : Inport  Port=2
  Constant : Constant  Value=p_b1
  Constant1 : Constant  Value=max_dist_from_ship
  Constant3 : Constant  Value=NED_psi_ds + pi
  Constant4 : Constant  Value=speed_0
  MATLAB Function1 : MATLAB Function  TreatAsAtomicUnit=on
  MATLAB Function7 : MATLAB Function  TreatAsAtomicUnit=on
  Memory : Memory
  speed : Outport
  yaw_dock : Outport  Port=2
  stop_flag : Outport  Port=3
  wires:
  MATLAB Function7/2(stop_flag) -> stop_flag/1, Memory/1
  Constant/1 -> MATLAB Function7/4(beacon)
  Memory/1 -> MATLAB Function7/1("stop_flag ")
  Constant3/1 -> MATLAB Function1/1("angle ")
  Constant1/1 -> MATLAB Function7/7(max_distance_from_ship)
  MATLAB Function1/1(angle) -> yaw_dock/1, MATLAB Function7/5(yaw_dock)
  Constant4/1 -> MATLAB Function7/6(speed_0)
  v_c/1 -> MATLAB Function7/2(v_c)
  p/1 -> MATLAB Function7/3(p)
  MATLAB Function7/1(speed) -> speed/1

== system simulation_environment_thesis/PHASE 1  /dynamic + kinematic models1 ==
  note: body frame
  note: ship frame
  note: ship frame
  tau : Inport
  ref speed : Inport  Port=2
  yaw prev : Inport  Port=3
  Constant : Constant  Value=0
  Demux3 : Demux
  Integrator : Integrator
  Integrator1 : Integrator  InitialCondition=const_current
  Integrator12 : Integrator  InitialConditionSource=external
  Integrator7 : Integrator  InitialConditionSource=external  InitialCondition=speed_0  UpperSaturationLimit=10^3  LowerSaturationLimit=-10^3
  MATLAB Function : MATLAB Function  TreatAsAtomicUnit=on
  MATLAB Function7 : MATLAB Function  TreatAsAtomicUnit=on
  MATLAB Function9 : MATLAB Function  TreatAsAtomicUnit=on
  Mux : Mux  Inputs=3
  Mux1 : Mux  Inputs=2
  Mux2 : Mux
  Random Number : RandomNumber  Variance=csi_x_1^2  Seed=75580913  SampleTime=dt
  Random Number1 : RandomNumber  Variance=csi_z_1^2  Seed=2046867146  SampleTime=dt
  Random Number10 : RandomNumber  Variance=csi_r_1^2  Seed=465862562  SampleTime=dt
  Random Number2 : RandomNumber  Variance=csi_vcz_1^2  Seed=1944131077  SampleTime=dt
  Random Number5 : RandomNumber  Variance=csi_y_1^2  Seed=116106138  SampleTime=dt
  Random Number6 : RandomNumber  Variance=csi_vcx_1^2  Seed=2146375792  SampleTime=dt
  Random Number7 : RandomNumber  Variance=csi_vcy_1^2  Seed=868631178  SampleTime=dt
  Random Number9 : RandomNumber  Variance=csi_psi_1^2  Seed=739183364  SampleTime=dt
  Selector : Selector  InputPortWidth=4  IndexOptions=Index vector (dialog)  Indices=[1 2 3]  OutputSizes=1
  Selector1 : Selector  InputPortWidth=4  IndexOptions=Index vector (dialog)  Indices=[4]  OutputSizes=1
  Selector2 : Selector  InputPortWidth=4  IndexOptions=Index vector (dialog)  Indices=[1 2 3]  OutputSizes=1
  Sum{,1..2,5} (4 alike) : Sum  Inputs=|++
  Sum4 : Sum  Inputs=|+-
  Unit Delay2 : UnitDelay  InitialCondition=position_t_0(4)  SampleTime=-1  HasFrameUpgradeWarning=on
  error speed : Outport
  v_tot inert : Outport  Port=2
  psi : Outport  Port=3
  p : Outport  Port=4
  body speed : Outport  Port=5
  wires:
  Integrator1/1 -> Sum5/2
  MATLAB Function7/1(speed_dot) -> Selector2/1, Selector1/1
  ref speed/1 -> Integrator7/2, Sum4/1
  Unit Delay2/1 -> Sum1/1, MATLAB Function9/1(yaw)
  Integrator12/1 -> Demux3/1
  MATLAB Function9/1(eta_dot) -> Integrator12/1, Sum5/1
  Sum4/1 -> error speed/1
  Sum5/1 -> Selector/1, v_tot inert/1
  yaw prev/1 -> Unit Delay2/1
  Integrator7/1 -> MATLAB Function7/1(speed), MATLAB Function9/2(speed), Sum4/2, body speed/1
  Demux3/4 -> MATLAB Function/1("angle ")
  tau/1 -> MATLAB Function7/2(tau)
  Integrator/1 -> p/1
  MATLAB Function/1(angle) -> psi/1
  Random Number10/1 -> Sum2/2
  Random Number7/1 -> Mux2/2
  Random Number6/1 -> Mux2/1
  Random Number9/1 -> Sum1/2
  Random Number/1 -> Mux/1
  Random Number5/1 -> Mux/2
  Sum/1 -> Integrator/1
  Mux/1 -> Sum/2
  Sum1/1 -> Integrator12/2
  Random Number1/1 -> Mux/3
  Selector1/1 -> Sum2/1
  Sum2/1 -> Mux1/2
  Selector2/1 -> Mux1/1
  Mux1/1 -> Integrator7/1
  Random Number2/1 -> Mux2/3
  Mux2/1 -> Integrator1/1
  Constant/1 -> Mux2/4
  Selector/1 -> Sum/1

== system simulation_environment_thesis/PHASE 1  /x,y,psi,r,vc estimate, XY PLANE from x,y,psi ==
  note: x_k_kprev
  note: x_knext_k
  note: ship frame
  note: [x
      | y]
  note: v
  note: doppler measurement noise
  note: u_k = v_m_k
  p : Inport
  inert_vel : Inport  Port=2
  psi : Inport  Port=3
  Constant : Constant  Value=C1
  Constant1 : Constant  Value=p_b1(1:2)
  Constant10 : Constant  Value=B1
  Constant12 : Constant  Value=const_current(1:2,1)  VectorParams1D=off
  Constant13 : Constant  Value=R1
  Constant14 : Constant  Value=A1
  Constant15 : Constant  Value=Q1
  Constant16 : Constant  Value=G1
  Constant9 : Constant  Value=A1  VectorParams1D=off
  Derivative : Derivative
  MATLAB Function : MATLAB Function  TreatAsAtomicUnit=on
  MATLAB Function10 : MATLAB Function  TreatAsAtomicUnit=on
  MATLAB Function6 : MATLAB Function  TreatAsAtomicUnit=on
  MATLAB Function7 : MATLAB Function  TreatAsAtomicUnit=on
  MATLAB Function8 : MATLAB Function  TreatAsAtomicUnit=on
  MATLAB Function9 : MATLAB Function  TreatAsAtomicUnit=on
  Mux : Mux  Inputs=3
  Mux1 : Mux  Inputs=2
  Mux2 : Mux  Inputs=2
  Mux3 : Mux  Inputs=2
  Random Number1 : RandomNumber  Variance=eta_x_1^2  Seed=1449464987  SampleTime=dt
  Random Number2 : RandomNumber  Variance=eta_y_1^2  Seed=2135957480  SampleTime=dt
  Random Number3 : RandomNumber  Variance=var_vm_x^2  Seed=1934193217  SampleTime=dt
  Random Number4 : RandomNumber  Variance=var_vm_y^2  Seed=2138077024  SampleTime=dt
  Random Number8 : RandomNumber  Variance=eta_psi_1^2  Seed=1986667015  SampleTime=dt
  Scope2 : Scope  NumInputPorts=5
  Selector : Selector  InputPortWidth=3  IndexOptions=Index vector (dialog)  Indices=[1 2]  OutputSizes=1
  Selector1 : Selector  InputPortWidth=6  IndexOptions=Index vector (dialog)  Indices=[1 2]  OutputSizes=1
  Selector2 : Selector  InputPortWidth=6  IndexOptions=Index vector (dialog)  Indices=[3 4 5 6]  OutputSizes=1
  Selector3 : Selector  InputPortWidth=6  IndexOptions=Index vector (dialog)  Indices=[3]  OutputSizes=1
  Selector4 : Selector  InputPortWidth=6  IndexOptions=Index vector (dialog)  Indices=[5 6]  OutputSizes=1
  Sum : Sum  Inputs=|++
  Sum5 : Sum  Inputs=|++
  Sum6 : Sum  Inputs=|+-
  Sum7 : Sum  Inputs=|++
  Sum8 : Sum  Inputs=|++-
  Unit Delay2 : UnitDelay  SampleTime=-1  HasFrameUpgradeWarning=on
  Unit Delay3 : UnitDelay  InitialCondition=alpha_xy_1*eye(6)  SampleTime=-1  HasFrameUpgradeWarning=on
  xy_est : Outport
  psi_est : Outport  Port=2
  v_c_xy : Outport  Port=3
  state_est : Outport  Port=4
  P_knext_knext : Outport  Port=5
  state_est_plane : Outport  Port=6
  wires:
  MATLAB Function7/1(x_k_k) -> Sum5/2
  Constant14/1 -> MATLAB Function10/3(A)
  Constant12/1 -> Sum8/3, Scope2/4
  Unit Delay2/1 -> Selector3/1, Selector4/1, MATLAB Function/1(u), state_est_plane/1, Selector2/1, Selector1/1, Scope2/5, Sum7/2, MATLAB Function6/1(x_k_kprev)
  Sum7/1 -> MATLAB Function7/2(u)
  Random Number4/1 -> Mux3/2
  MATLAB Function8/1(y) -> Sum5/1
  Random Number3/1 -> Mux3/1
  Constant15/1 -> MATLAB Function10/4(Q)
  MATLAB Function9/1(y) -> Sum7/1
  MATLAB Function10/2(P_knext_knext) -> Unit Delay3/1, P_knext_knext/1
  Sum8/1 -> MATLAB Function8/2(u)
  MATLAB Function10/1(K_knext) -> MATLAB Function9/1(K)
  Selector/1 -> Mux1/1, Scope2/1
  Constant9/1 -> MATLAB Function7/1(A)
  Constant13/1 -> MATLAB Function10/2(R)
  Sum6/1 -> MATLAB Function9/2(y_err)
  Constant10/1 -> MATLAB Function8/1(B)
  Unit Delay3/1 -> MATLAB Function10/1(P_k_k)
  Mux3/1 -> Sum8/1
  Constant16/1 -> MATLAB Function10/5(G)
  inert_vel/1 -> Sum8/2
  Sum5/1 -> Unit Delay2/1
  p/1 -> Selector/1
  Mux/1 -> Sum/2
  Sum/1 -> Sum6/1
  MATLAB Function6/1(pred) -> Sum6/2
  Constant/1 -> MATLAB Function6/2(C), MATLAB Function10/6(C)
  Selector1/1 -> xy_est/1
  psi/1 -> Derivative/1, Scope2/2, Mux1/2
  Mux1/1 -> Sum/1
  Selector3/1 -> psi_est/1
  Selector4/1 -> v_c_xy/1
  Derivative/1 -> Scope2/3
  Random Number2/1 -> Mux/2
  Random Number8/1 -> Mux/3
  Random Number1/1 -> Mux/1
  Constant1/1 -> MATLAB Function/2(ship_p_ds)
  MATLAB Function/1(y) -> Mux2/1
  Selector2/1 -> Mux2/2
  Mux2/1 -> state_est/1

== system simulation_environment_thesis/PHASE 1  /z, v_c_z estimate from DEPTH measurement ==
  note: v
  note: u_k = v_m_k
  note: z_m
  p : Inport
  Constant : Constant  Value=p_b1(3)
  Constant10 : Constant  Value=Az1
  Constant11 : Constant  Value=Bz1
  Constant12 : Constant  Value=Cz1  VectorParams1D=off
  Constant13 : Constant  Value=const_current(3,1)
  Constant14 : Constant  Value=Rz1
  Constant15 : Constant  Value=Az1
  Constant16 : Constant  Value=Qz1
  Constant17 : Constant  Value=Gz1
  Constant9 : Constant  Value=Cz1  VectorParams1D=off
  Demux : Demux  Outputs=2
  Derivative1 : Derivative
  MATLAB Function : MATLAB Function  TreatAsAtomicUnit=on
  MATLAB Function1 : MATLAB Function  TreatAsAtomicUnit=on
  MATLAB Function6 : MATLAB Function  TreatAsAtomicUnit=on
  MATLAB Function7 : MATLAB Function  TreatAsAtomicUnit=on
  MATLAB Function8 : MATLAB Function  TreatAsAtomicUnit=on
  MATLAB Function9 : MATLAB Function  TreatAsAtomicUnit=on
  Mux1 : Mux  Inputs=2
  Random Number1 : RandomNumber  Variance=var_vm_z^2  Seed=1691368948  SampleTime=dt
  Scope2 : Scope  NumInputPorts=3
  Scope3 : Scope
  Selector : Selector  InputPortWidth=2  IndexOptions=Index vector (dialog)  Indices=[2]  OutputSizes=1
  Selector1 : Selector  InputPortWidth=3  IndexOptions=Index vector (dialog)  Indices=[3]  OutputSizes=1
  Sum5 : Sum  Inputs=|++
  Sum6 : Sum  Inputs=|+-
  Sum7 : Sum  Inputs=|++
  Sum8 : Sum  Inputs=|++-
  Sum9 : Sum  Inputs=|++
  Unit Delay2 : UnitDelay  SampleTime=-1  HasFrameUpgradeWarning=on
  Unit Delay3 : UnitDelay  InitialCondition=alpha_z_1*eye(2)  SampleTime=-1  HasFrameUpgradeWarning=on
  eta3 : RandomNumber  Variance=eta_z_1^2  Seed=43842239  SampleTime=dt
  z_est : Outport
  state_est : Outport  Port=2
  P_k_k : Outport  Port=3
  state_est_z : Outport  Port=4
  wires:
  Derivative1/1 -> Sum8/2
  Constant11/1 -> MATLAB Function7/1(B)
  Selector1/1 -> Derivative1/1, Scope2/1, Sum9/1
  Constant16/1 -> MATLAB Function9/5(Q)
  Demux/1 -> z_est/1
  MATLAB Function9/2(P_k_k) -> Unit Delay3/1, P_k_k/1
  Constant14/1 -> MATLAB Function9/3(R)
  Unit Delay2/1 -> Demux/1, Scope2/2, state_est_z/1, Selector/1, MATLAB Function/1(u), Sum7/2, MATLAB Function1/2(u)
  Constant12/1 -> MATLAB Function9/2(C)
  MATLAB Function1/1(y) -> Sum6/2
  MATLAB Function6/1(y) -> Sum5/2
  Sum7/1 -> MATLAB Function6/2(u)
  Random Number1/1 -> Sum8/1
  Sum6/1 -> MATLAB Function8/2(u)
  Constant9/1 -> MATLAB Function1/1(C)
  MATLAB Function9/1(K_k) -> Scope3/1, MATLAB Function8/1(K)
  Sum5/1 -> Unit Delay2/1
  Sum8/1 -> MATLAB Function7/2(u)
  Constant13/1 -> Sum8/3, Scope2/3
  MATLAB Function7/1(y) -> Sum5/1
  Sum9/1 -> Sum6/1
  Constant10/1 -> MATLAB Function6/1(A)
  MATLAB Function8/1(y) -> Sum7/1
  Constant15/1 -> MATLAB Function9/4(A)
  Constant17/1 -> MATLAB Function9/6(G)
  Unit Delay3/1 -> MATLAB Function9/1("P_k_k ")
  eta3/1 -> Sum9/2
  p/1 -> Selector1/1
  Constant/1 -> MATLAB Function/2(ship_p_ds)
  MATLAB Function/1(y) -> Mux1/1
  Selector/1 -> Mux1/2
  Mux1/1 -> state_est/1

== system simulation_environment_thesis/PHASE 1.21/CONTROLLER1 ==
  v_err : Inport
  speed_0 : Inport  Port=2
  yaw_err : Inport  Port=3
  z_err : Inport  Port=4
  ANTIWINDUP SURGE : Subsystem
  ANTIWINDUP SWAY : Subsystem
  ANTIWINDUP_HEAVE : Subsystem
  ANTIWINDUP_YAW : Subsystem
  Demux : Demux
  MATLAB Function3 : MATLAB Function  TreatAsAtomicUnit=on
  Mux : Mux
  tau : Outport
  wires:
  ANTIWINDUP SURGE/1(tau_u) -> Mux/1
  ANTIWINDUP_HEAVE/1(tau_w) -> Mux/3
  MATLAB Function3/1(K_i_u) -> ANTIWINDUP SURGE/2(K)
  MATLAB Function3/3(K_t_u) -> ANTIWINDUP SURGE/4(K_t_u)
  MATLAB Function3/2(K_p_u) -> ANTIWINDUP SURGE/3(K1)
  speed_0/1 -> MATLAB Function3/1(speed_0)
  Demux/1 -> ANTIWINDUP SURGE/1(in)
  ANTIWINDUP SWAY/1(tau_v) -> Mux/2
  v_err/1 -> Demux/1
  Mux/1 -> tau/1
  Demux/2 -> ANTIWINDUP SWAY/1(in)
  MATLAB Function3/4(K_i_v) -> ANTIWINDUP SWAY/2(K)
  MATLAB Function3/5(K_p_v) -> ANTIWINDUP SWAY/3(K1)
  MATLAB Function3/6(K_t_v) -> ANTIWINDUP SWAY/4(K_t_v)
  ANTIWINDUP_YAW/1(tau_psi) -> Mux/4
  MATLAB Function3/14(K_t_psi) -> ANTIWINDUP_YAW/3(K_t_psi)
  MATLAB Function3/13(K_d_psi) -> ANTIWINDUP_YAW/6(K)
  Demux/4 -> ANTIWINDUP_YAW/5(in)
  MATLAB Function3/12(K_p_psi) -> ANTIWINDUP_YAW/2(K2)
  MATLAB Function3/11(K_i_psi) -> ANTIWINDUP_YAW/1(K1)
  yaw_err/1 -> ANTIWINDUP_YAW/4(in1)
  MATLAB Function3/7(K_i_w) -> ANTIWINDUP_HEAVE/1(K1)
  MATLAB Function3/8(K_p_w) -> ANTIWINDUP_HEAVE/2(K2)
  MATLAB Function3/10(K_t_w) -> ANTIWINDUP_HEAVE/3(K_t_w)
  MATLAB Function3/9(K_d_w) -> ANTIWINDUP_HEAVE/6(K)
  z_err/1 -> ANTIWINDUP_HEAVE/4(in1)
  Demux/3 -> ANTIWINDUP_HEAVE/5(in)

== system simulation_environment_thesis/PHASE 1.21/dynamic + kinematic models3 ==
  note: body frame
  note: ship frame
  note: ship frame
  tau : Inport
  ref speed : Inport  Port=2
  yaw prev : Inport  Port=3
  Constant : Constant  Value=0
  Demux3 : Demux
  Integrator : Integrator
  Integrator1 : Integrator  InitialCondition=const_current
  Integrator12 : Integrator  InitialConditionSource=external
  Integrator7 : Integrator  InitialCondition=speed_0  UpperSaturationLimit=10^3  LowerSaturationLimit=-10^3
  MATLAB Function : MATLAB Function  TreatAsAtomicUnit=on
  MATLAB Function7 : MATLAB Function  TreatAsAtomicUnit=on
  MATLAB Function9 : MATLAB Function  TreatAsAtomicUnit=on
  Mux : Mux  Inputs=3
  Mux1 : Mux  Inputs=2
  Mux2 : Mux
  Random Number : RandomNumber  Variance=csi_x_1^2  Seed=2028119420  SampleTime=dt
  Random Number1 : RandomNumber  Variance=csi_z_1^2  Seed=120715227  SampleTime=dt
  Random Number10 : RandomNumber  Variance=csi_r_1^2  Seed=1310558832  SampleTime=dt
  Random Number2 : RandomNumber  Variance=csi_vcz_1^2  Seed=1389698884  SampleTime=dt
  Random Number5 : RandomNumber  Variance=csi_y_1^2  Seed=316193166  SampleTime=dt
  Random Number6 : RandomNumber  Variance=csi_vcx_1^2  Seed=1546237725  SampleTime=dt
  Random Number7 : RandomNumber  Variance=csi_vcy_1^2  Seed=1951515759  SampleTime=dt
  Random Number9 : RandomNumber  Variance=csi_psi_1^2  Seed=1481719397  SampleTime=dt
  Selector : Selector  InputPortWidth=4  IndexOptions=Index vector (dialog)  Indices=[1 2 3]  OutputSizes=1
  Selector1 : Selector  InputPortWidth=4  IndexOptions=Index vector (dialog)  Indices=[4]  OutputSizes=1
  Selector2 : Selector  InputPortWidth=4  IndexOptions=Index vector (dialog)  Indices=[1 2 3]  OutputSizes=1
  Sum{,1..2,5} (4 alike) : Sum  Inputs=|++
  Sum4 : Sum  Inputs=|+-
  Unit Delay2 : UnitDelay  InitialCondition=position_t_0(4)  SampleTime=-1  HasFrameUpgradeWarning=on
  error speed : Outport
  v_tot inert : Outport  Port=2
  psi : Outport  Port=3
  p : Outport  Port=4
  body speed : Outport  Port=5
  wires:
  Integrator1/1 -> Sum5/2
  MATLAB Function7/1(speed_dot) -> Selector2/1, Selector1/1
  ref speed/1 -> Sum4/1
  Unit Delay2/1 -> Sum1/1, MATLAB Function9/1(yaw)
  Integrator12/1 -> Demux3/1
  MATLAB Function9/1(eta_dot) -> Integrator12/1, Sum5/1
  Sum4/1 -> error speed/1
  Sum5/1 -> Selector/1, v_tot inert/1
  yaw prev/1 -> Unit Delay2/1
  Integrator7/1 -> MATLAB Function9/2(speed), Sum4/2, body speed/1, MATLAB Function7/1(speed)
  Demux3/4 -> MATLAB Function/1("angle ")
  tau/1 -> MATLAB Function7/2(tau)
  Integrator/1 -> p/1
  MATLAB Function/1(angle) -> psi/1
  Random Number10/1 -> Sum2/2
  Random Number7/1 -> Mux2/2
  Random Number6/1 -> Mux2/1
  Random Number9/1 -> Sum1/2
  Random Number/1 -> Mux/1
  Random Number5/1 -> Mux/2
  Sum/1 -> Integrator/1
  Mux/1 -> Sum/2
  Sum1/1 -> Integrator12/2
  Random Number1/1 -> Mux/3
  Selector1/1 -> Sum2/1
  Sum2/1 -> Mux1/2
  Selector2/1 -> Mux1/1
  Mux1/1 -> Integrator7/1
  Random Number2/1 -> Mux2/3
  Mux2/1 -> Integrator1/1
  Constant/1 -> Mux2/4
  Selector/1 -> Sum/1
